simulink model "wlan_phy_rx_pmd"  (Simulink 7.8)
settings: Solver=VariableStepDiscrete  SolverMode=SingleTasking  StartTime=0.0  StopTime=rx_sim.sim_time  RelTol=1e-3
PostLoadFcn:
  | wlan_phy_rx_init
InitFcn:
  | wlan_phy_rx_init
StartFcn:
  | wlan_phy_rx_init
values from neighbouring files (symbols this model uses):
  barker_seq20 = 0.95 * circshift(barker_seq20, [0 4]) ./ max(abs(barker_seq20))    [wlan_phy_rx_init.m]
  bit_scrambler_lfsr_bytes = sum(bit_scrambler_lfsr_bytes .* repmat(2.^[0:7], 127, 1),2)    [wlan_phy_rx_init.m]
  longCorr_coef_i = [3*real(longCorr_coef)]    [wlan_phy_rx_init.m]
  longCorr_coef_q = [3*imag(longCorr_coef)]    [wlan_phy_rx_init.m]
  MAX_CP_LEN = 32    [wlan_phy_rx_init.m]
  MAX_NCBPS = NCBPS_11n_64QAM    (= 312)    [wlan_phy_rx_init.m]
  MAX_NUM_BYTES = 2^16-1    (= 65535)    [wlan_phy_rx_init.m]
  MAX_NUM_SC = 64    [wlan_phy_rx_init.m]
  MAX_NUM_SYMS = 600    [wlan_phy_rx_init.m]
  NDBPS_11a_BPSK12 = 24    [wlan_phy_rx_init.m]
  preFFT_sampBuff_numSamps = 4*MAX_NUM_SC    (= 256)    [wlan_phy_rx_init.m]
  rx_sim = struct()    [wlan_phy_rx_init.m]
  scr_ind_rev = zeros(1,128)    [wlan_phy_rx_init.m]
undefined symbols (not defined in the file or in its folder): gcbh, mcs_rom_11ag, mcs_rom_11n, wlan_rx_deinterleave_rom_gen, xlBlockLoadCallback, xlGetNormalizedPeriod, xlipgetpartsetting
libraries: xbsIndex_r4 x4943, xbsEDKLib_r4 x1
(part 21 of 28)

== system wlan_phy_rx_pmd/Sync & Antenna Sel/LTS Correlation/Correlator/C4/C4 ==
  x : Inport
  vin : Inport  Port=2
  "  " : Inport  Port=3
  "     " : Inport  Port=4
  ASR : xbsIndex_r4/Addressable Shift Register  infer_latency=off  depth=length(coef2)  initVector=[0]  en=on  dbl_ovrd=off  use_behavioral_HDL=off  Optimization=Resource  use_rpm=off  block_type=addrsr
      settings: infoedit="Delay of configurable length.  Any element in the delay line can be addressed and driven onto the output port.<br><br>Hardware notes: This block is implemented using SRL16s. If Virtex-4 or Spartan-3 device is used, multiple SRLC16s are cascaded together."  xl_area=[1 0 0 2 0 0 0]  block_version=VER_STRING_GOES_HERE
  Delay : xbsIndex_r4/Delay  rst=off  infoeditControl=Selection of Reset will increase slice count due to use of real FFs and instead of SRLs  en=off  latency=1  dbl_ovrd=off  reg_retiming=off  block_type=delay
  Gateway Out{1..8} (8 alike) : xbsIndex_r4/Gateway Out  inherit_from_input=off  hdl_port=off  timing_constraint=None  locs_specified=off  LOCs={}  UseAsDAC=off  DACChannel='1'  block_type=gatewayout
      settings: infoedit="Gateway out block.  Converts Xilinx fixed point inputs into ouputs of type Simulink integer, double, or fixed point.<P><P>Hardware notes:  In hardware these blocks become top level output ports or are discarded, depending on how they are configured."  xl_area=[0 0 0 0 0 0 0]  block_version=VER_STRING_GOES_HERE
  MAC : Subsystem
  ROM : xbsIndex_r4/ROM  depth=length(coef2)  initVector=coef2  distributed_mem=Distributed memory  rst=off  init_reg=0  en=off  latency=1  gui_display_data_type=Fixed-point  arith_type="Signed  (2's comp)"  n_bits=longCorr_coef_nbits  bin_pt=longCorr_coef_bp  preci_type=Single  exp_width=8  frac_width=24  dbl_ovrd=off  optimize=Area  use_rpm=off  block_type=sprom
      settings: xl_area=[1 1 0 1 0 0 0]  block_version=VER_STRING_GOES_HERE
  x_dly : Outport
  x_dly_vout : Outport  Port=2
  y : Outport  Port=3
  y_vout : Outport  Port=4
  wires:
  Gateway Out5/1 -> ?  "ROM Addr"
  Gateway Out4/1 -> ?  "Corr Out"
  Gateway Out3/1 -> ?  "Corr Ld"
  Gateway Out2/1 -> ?  "ROM Out"
  Gateway Out1/1 -> ?  "ASR Out"
  Gateway Out6/1 -> ?  "X In"
  Gateway Out7/1 -> ?  "ASR Addr"
  Gateway Out8/1 -> ?  "X Vin"
  Delay/1 -> y_vout/1
  vin/1 -> x_dly_vout/1, MAC/4(rst), Gateway Out8/1, ASR/3
  x/1 -> Gateway Out6/1, ASR/1
  [ltsCorr_dAddr] -> Gateway Out7/1, ASR/2
  [ltsCorr_cAddr] -> Gateway Out5/1, ROM/1
  ASR/1 -> Gateway Out1/1, x_dly/1, MAC/1(a)
  MAC/1(yn) -> Gateway Out4/1, y/1
  ROM/1 -> Gateway Out2/1, MAC/2(coeff)
  [ltsCorr_ld] -> Gateway Out3/1, Delay/1, MAC/3(ld)

== system wlan_phy_rx_pmd/Sync & Antenna Sel/LTS Correlation/Correlator/C4/C5 ==
  x : Inport
  vin : Inport  Port=2
  "  " : Inport  Port=3
  "     " : Inport  Port=4
  ASR : xbsIndex_r4/Addressable Shift Register  infer_latency=off  depth=length(coef2)  initVector=[0]  en=on  dbl_ovrd=off  use_behavioral_HDL=off  Optimization=Resource  use_rpm=off  block_type=addrsr
      settings: infoedit="Delay of configurable length.  Any element in the delay line can be addressed and driven onto the output port.<br><br>Hardware notes: This block is implemented using SRL16s. If Virtex-4 or Spartan-3 device is used, multiple SRLC16s are cascaded together."  xl_area=[1 0 0 2 0 0 0]  block_version=VER_STRING_GOES_HERE
  Delay : xbsIndex_r4/Delay  rst=off  infoeditControl=Selection of Reset will increase slice count due to use of real FFs and instead of SRLs  en=off  latency=1  dbl_ovrd=off  reg_retiming=off  block_type=delay
  Gateway Out{1..8} (8 alike) : xbsIndex_r4/Gateway Out  inherit_from_input=off  hdl_port=off  timing_constraint=None  locs_specified=off  LOCs={}  UseAsDAC=off  DACChannel='1'  block_type=gatewayout
      settings: infoedit="Gateway out block.  Converts Xilinx fixed point inputs into ouputs of type Simulink integer, double, or fixed point.<P><P>Hardware notes:  In hardware these blocks become top level output ports or are discarded, depending on how they are configured."  xl_area=[0 0 0 0 0 0 0]  block_version=VER_STRING_GOES_HERE
  MAC : Subsystem
  ROM : xbsIndex_r4/ROM  depth=length(coef2)  initVector=coef2  distributed_mem=Distributed memory  rst=off  init_reg=0  en=off  latency=1  gui_display_data_type=Fixed-point  arith_type="Signed  (2's comp)"  n_bits=longCorr_coef_nbits  bin_pt=longCorr_coef_bp  preci_type=Single  exp_width=8  frac_width=24  dbl_ovrd=off  optimize=Area  use_rpm=off  block_type=sprom
      settings: xl_area=[1 1 0 1 0 0 0]  block_version=VER_STRING_GOES_HERE
  x_dly : Outport
  x_dly_vout : Outport  Port=2
  y : Outport  Port=3
  y_vout : Outport  Port=4
  wires:
  [ltsCorr_ld] -> MAC/3(ld), Delay/1, Gateway Out3/1
  ROM/1 -> MAC/2(coeff), Gateway Out2/1
  MAC/1(yn) -> y/1, Gateway Out4/1
  ASR/1 -> MAC/1(a), x_dly/1, Gateway Out1/1
  [ltsCorr_cAddr] -> ROM/1, Gateway Out5/1
  [ltsCorr_dAddr] -> ASR/2, Gateway Out7/1
  x/1 -> ASR/1, Gateway Out6/1
  vin/1 -> ASR/3, Gateway Out8/1, MAC/4(rst), x_dly_vout/1
  Delay/1 -> y_vout/1
  Gateway Out8/1 -> ?  "X Vin"
  Gateway Out7/1 -> ?  "ASR Addr"
  Gateway Out6/1 -> ?  "X In"
  Gateway Out1/1 -> ?  "ASR Out"
  Gateway Out2/1 -> ?  "ROM Out"
  Gateway Out3/1 -> ?  "Corr Ld"
  Gateway Out4/1 -> ?  "Corr Out"
  Gateway Out5/1 -> ?  "ROM Addr"

== system wlan_phy_rx_pmd/Sync & Antenna Sel/LTS Correlation/Correlator/C4/C6 ==
  x : Inport
  vin : Inport  Port=2
  "  " : Inport  Port=3
  "     " : Inport  Port=4
  ASR : xbsIndex_r4/Addressable Shift Register  infer_latency=off  depth=length(coef2)  initVector=[0]  en=on  dbl_ovrd=off  use_behavioral_HDL=off  Optimization=Resource  use_rpm=off  block_type=addrsr
      settings: infoedit="Delay of configurable length.  Any element in the delay line can be addressed and driven onto the output port.<br><br>Hardware notes: This block is implemented using SRL16s. If Virtex-4 or Spartan-3 device is used, multiple SRLC16s are cascaded together."  xl_area=[1 0 0 2 0 0 0]  block_version=VER_STRING_GOES_HERE
  Delay : xbsIndex_r4/Delay  rst=off  infoeditControl=Selection of Reset will increase slice count due to use of real FFs and instead of SRLs  en=off  latency=1  dbl_ovrd=off  reg_retiming=off  block_type=delay
  Gateway Out{1..8} (8 alike) : xbsIndex_r4/Gateway Out  inherit_from_input=off  hdl_port=off  timing_constraint=None  locs_specified=off  LOCs={}  UseAsDAC=off  DACChannel='1'  block_type=gatewayout
      settings: infoedit="Gateway out block.  Converts Xilinx fixed point inputs into ouputs of type Simulink integer, double, or fixed point.<P><P>Hardware notes:  In hardware these blocks become top level output ports or are discarded, depending on how they are configured."  xl_area=[0 0 0 0 0 0 0]  block_version=VER_STRING_GOES_HERE
  MAC : Subsystem
  ROM : xbsIndex_r4/ROM  depth=length(coef2)  initVector=coef2  distributed_mem=Distributed memory  rst=off  init_reg=0  en=off  latency=1  gui_display_data_type=Fixed-point  arith_type="Signed  (2's comp)"  n_bits=longCorr_coef_nbits  bin_pt=longCorr_coef_bp  preci_type=Single  exp_width=8  frac_width=24  dbl_ovrd=off  optimize=Area  use_rpm=off  block_type=sprom
      settings: xl_area=[1 1 0 1 0 0 0]  block_version=VER_STRING_GOES_HERE
  x_dly : Outport
  x_dly_vout : Outport  Port=2
  y : Outport  Port=3
  y_vout : Outport  Port=4
  wires:
  Gateway Out5/1 -> ?  "ROM Addr"
  Gateway Out4/1 -> ?  "Corr Out"
  Gateway Out3/1 -> ?  "Corr Ld"
  Gateway Out2/1 -> ?  "ROM Out"
  Gateway Out1/1 -> ?  "ASR Out"
  Gateway Out6/1 -> ?  "X In"
  Gateway Out7/1 -> ?  "ASR Addr"
  Gateway Out8/1 -> ?  "X Vin"
  Delay/1 -> y_vout/1
  vin/1 -> x_dly_vout/1, MAC/4(rst), Gateway Out8/1, ASR/3
  x/1 -> Gateway Out6/1, ASR/1
  [ltsCorr_dAddr] -> Gateway Out7/1, ASR/2
  [ltsCorr_cAddr] -> Gateway Out5/1, ROM/1
  ASR/1 -> Gateway Out1/1, x_dly/1, MAC/1(a)
  MAC/1(yn) -> Gateway Out4/1, y/1
  ROM/1 -> Gateway Out2/1, MAC/2(coeff)
  [ltsCorr_ld] -> Gateway Out3/1, Delay/1, MAC/3(ld)

== system wlan_phy_rx_pmd/Sync & Antenna Sel/LTS Correlation/Correlator/C4/C7 ==
  x : Inport
  vin : Inport  Port=2
  "  " : Inport  Port=3
  "     " : Inport  Port=4
  ASR : xbsIndex_r4/Addressable Shift Register  infer_latency=off  depth=length(coef2)  initVector=[0]  en=on  dbl_ovrd=off  use_behavioral_HDL=off  Optimization=Resource  use_rpm=off  block_type=addrsr
      settings: infoedit="Delay of configurable length.  Any element in the delay line can be addressed and driven onto the output port.<br><br>Hardware notes: This block is implemented using SRL16s. If Virtex-4 or Spartan-3 device is used, multiple SRLC16s are cascaded together."  xl_area=[1 0 0 2 0 0 0]  block_version=VER_STRING_GOES_HERE
  Delay : xbsIndex_r4/Delay  rst=off  infoeditControl=Selection of Reset will increase slice count due to use of real FFs and instead of SRLs  en=off  latency=1  dbl_ovrd=off  reg_retiming=off  block_type=delay
  Gateway Out{1..8} (8 alike) : xbsIndex_r4/Gateway Out  inherit_from_input=off  hdl_port=off  timing_constraint=None  locs_specified=off  LOCs={}  UseAsDAC=off  DACChannel='1'  block_type=gatewayout
      settings: infoedit="Gateway out block.  Converts Xilinx fixed point inputs into ouputs of type Simulink integer, double, or fixed point.<P><P>Hardware notes:  In hardware these blocks become top level output ports or are discarded, depending on how they are configured."  xl_area=[0 0 0 0 0 0 0]  block_version=VER_STRING_GOES_HERE
  MAC : Subsystem
  ROM : xbsIndex_r4/ROM  depth=length(coef2)  initVector=coef2  distributed_mem=Distributed memory  rst=off  init_reg=0  en=off  latency=1  gui_display_data_type=Fixed-point  arith_type="Signed  (2's comp)"  n_bits=longCorr_coef_nbits  bin_pt=longCorr_coef_bp  preci_type=Single  exp_width=8  frac_width=24  dbl_ovrd=off  optimize=Area  use_rpm=off  block_type=sprom
      settings: xl_area=[1 1 0 1 0 0 0]  block_version=VER_STRING_GOES_HERE
  x_dly : Outport
  x_dly_vout : Outport  Port=2
  y : Outport  Port=3
  y_vout : Outport  Port=4
  wires:
  [ltsCorr_ld] -> MAC/3(ld), Delay/1, Gateway Out3/1
  ROM/1 -> MAC/2(coeff), Gateway Out2/1
  MAC/1(yn) -> y/1, Gateway Out4/1
  ASR/1 -> MAC/1(a), x_dly/1, Gateway Out1/1
  [ltsCorr_cAddr] -> ROM/1, Gateway Out5/1
  [ltsCorr_dAddr] -> ASR/2, Gateway Out7/1
  x/1 -> ASR/1, Gateway Out6/1
  vin/1 -> ASR/3, Gateway Out8/1, MAC/4(rst), x_dly_vout/1
  Delay/1 -> y_vout/1
  Gateway Out8/1 -> ?  "X Vin"
  Gateway Out7/1 -> ?  "ASR Addr"
  Gateway Out6/1 -> ?  "X In"
  Gateway Out1/1 -> ?  "ASR Out"
  Gateway Out2/1 -> ?  "ROM Out"
  Gateway Out3/1 -> ?  "Corr Ld"
  Gateway Out4/1 -> ?  "Corr Out"
  Gateway Out5/1 -> ?  "ROM Addr"

== system wlan_phy_rx_pmd/Sync & Antenna Sel/LTS Correlation/Correlator/C4/C8 ==
  x : Inport
  vin : Inport  Port=2
  "  " : Inport  Port=3
  "     " : Inport  Port=4
  ASR : xbsIndex_r4/Addressable Shift Register  infer_latency=off  depth=length(coef2)  initVector=[0]  en=on  dbl_ovrd=off  use_behavioral_HDL=off  Optimization=Resource  use_rpm=off  block_type=addrsr
      settings: infoedit="Delay of configurable length.  Any element in the delay line can be addressed and driven onto the output port.<br><br>Hardware notes: This block is implemented using SRL16s. If Virtex-4 or Spartan-3 device is used, multiple SRLC16s are cascaded together."  xl_area=[1 0 0 2 0 0 0]  block_version=VER_STRING_GOES_HERE
  Delay : xbsIndex_r4/Delay  rst=off  infoeditControl=Selection of Reset will increase slice count due to use of real FFs and instead of SRLs  en=off  latency=1  dbl_ovrd=off  reg_retiming=off  block_type=delay
  Gateway Out{1..8} (8 alike) : xbsIndex_r4/Gateway Out  inherit_from_input=off  hdl_port=off  timing_constraint=None  locs_specified=off  LOCs={}  UseAsDAC=off  DACChannel='1'  block_type=gatewayout
      settings: infoedit="Gateway out block.  Converts Xilinx fixed point inputs into ouputs of type Simulink integer, double, or fixed point.<P><P>Hardware notes:  In hardware these blocks become top level output ports or are discarded, depending on how they are configured."  xl_area=[0 0 0 0 0 0 0]  block_version=VER_STRING_GOES_HERE
  MAC : Subsystem
  ROM : xbsIndex_r4/ROM  depth=length(coef2)  initVector=coef2  distributed_mem=Distributed memory  rst=off  init_reg=0  en=off  latency=1  gui_display_data_type=Fixed-point  arith_type="Signed  (2's comp)"  n_bits=longCorr_coef_nbits  bin_pt=longCorr_coef_bp  preci_type=Single  exp_width=8  frac_width=24  dbl_ovrd=off  optimize=Area  use_rpm=off  block_type=sprom
      settings: xl_area=[1 1 0 1 0 0 0]  block_version=VER_STRING_GOES_HERE
  x_dly : Outport
  x_dly_vout : Outport  Port=2
  y : Outport  Port=3
  y_vout : Outport  Port=4
  wires:
  Gateway Out5/1 -> ?  "ROM Addr"
  Gateway Out4/1 -> ?  "Corr Out"
  Gateway Out3/1 -> ?  "Corr Ld"
  Gateway Out2/1 -> ?  "ROM Out"
  Gateway Out1/1 -> ?  "ASR Out"
  Gateway Out6/1 -> ?  "X In"
  Gateway Out7/1 -> ?  "ASR Addr"
  Gateway Out8/1 -> ?  "X Vin"
  Delay/1 -> y_vout/1
  vin/1 -> x_dly_vout/1, MAC/4(rst), Gateway Out8/1, ASR/3
  x/1 -> Gateway Out6/1, ASR/1
  [ltsCorr_dAddr] -> Gateway Out7/1, ASR/2
  [ltsCorr_cAddr] -> Gateway Out5/1, ROM/1
  ASR/1 -> Gateway Out1/1, x_dly/1, MAC/1(a)
  MAC/1(yn) -> Gateway Out4/1, y/1
  ROM/1 -> Gateway Out2/1, MAC/2(coeff)
  [ltsCorr_ld] -> Gateway Out3/1, Delay/1, MAC/3(ld)

== system wlan_phy_rx_pmd/Sync & Antenna Sel/LTS Correlation/Correlator/C5/C1 ==
  x : Inport
  vin : Inport  Port=2
  "  " : Inport  Port=3
  "     " : Inport  Port=4
  ASR : xbsIndex_r4/Addressable Shift Register  infer_latency=off  depth=length(coef2)  initVector=[0]  en=on  dbl_ovrd=off  use_behavioral_HDL=off  Optimization=Resource  use_rpm=off  block_type=addrsr
      settings: infoedit="Delay of configurable length.  Any element in the delay line can be addressed and driven onto the output port.<br><br>Hardware notes: This block is implemented using SRL16s. If Virtex-4 or Spartan-3 device is used, multiple SRLC16s are cascaded together."  xl_area=[1 0 0 2 0 0 0]  block_version=VER_STRING_GOES_HERE
  Delay : xbsIndex_r4/Delay  rst=off  infoeditControl=Selection of Reset will increase slice count due to use of real FFs and instead of SRLs  en=off  latency=1  dbl_ovrd=off  reg_retiming=off  block_type=delay
  Gateway Out{1..8} (8 alike) : xbsIndex_r4/Gateway Out  inherit_from_input=off  hdl_port=off  timing_constraint=None  locs_specified=off  LOCs={}  UseAsDAC=off  DACChannel='1'  block_type=gatewayout
      settings: infoedit="Gateway out block.  Converts Xilinx fixed point inputs into ouputs of type Simulink integer, double, or fixed point.<P><P>Hardware notes:  In hardware these blocks become top level output ports or are discarded, depending on how they are configured."  xl_area=[0 0 0 0 0 0 0]  block_version=VER_STRING_GOES_HERE
  MAC : Subsystem
  ROM : xbsIndex_r4/ROM  depth=length(coef2)  initVector=coef2  distributed_mem=Distributed memory  rst=off  init_reg=0  en=off  latency=1  gui_display_data_type=Fixed-point  arith_type="Signed  (2's comp)"  n_bits=longCorr_coef_nbits  bin_pt=longCorr_coef_bp  preci_type=Single  exp_width=8  frac_width=24  dbl_ovrd=off  optimize=Area  use_rpm=off  block_type=sprom
      settings: xl_area=[1 1 0 1 0 0 0]  block_version=VER_STRING_GOES_HERE
  x_dly : Outport
  x_dly_vout : Outport  Port=2
  y : Outport  Port=3
  y_vout : Outport  Port=4
  wires:
  Gateway Out5/1 -> ?  "ROM Addr"
  Gateway Out4/1 -> ?  "Corr Out"
  Gateway Out3/1 -> ?  "Corr Ld"
  Gateway Out2/1 -> ?  "ROM Out"
  Gateway Out1/1 -> ?  "ASR Out"
  Gateway Out6/1 -> ?  "X In"
  Gateway Out7/1 -> ?  "ASR Addr"
  Gateway Out8/1 -> ?  "X Vin"
  Delay/1 -> y_vout/1
  vin/1 -> x_dly_vout/1, MAC/4(rst), Gateway Out8/1, ASR/3
  x/1 -> Gateway Out6/1, ASR/1
  [ltsCorr_dAddr] -> Gateway Out7/1, ASR/2
  [ltsCorr_cAddr] -> Gateway Out5/1, ROM/1
  ASR/1 -> Gateway Out1/1, x_dly/1, MAC/1(a)
  MAC/1(yn) -> Gateway Out4/1, y/1
  ROM/1 -> Gateway Out2/1, MAC/2(coeff)
  [ltsCorr_ld] -> Gateway Out3/1, Delay/1, MAC/3(ld)

== system wlan_phy_rx_pmd/Sync & Antenna Sel/LTS Correlation/Correlator/C5/C2 ==
  x : Inport
  vin : Inport  Port=2
  "  " : Inport  Port=3
  "     " : Inport  Port=4
  ASR : xbsIndex_r4/Addressable Shift Register  infer_latency=off  depth=length(coef2)  initVector=[0]  en=on  dbl_ovrd=off  use_behavioral_HDL=off  Optimization=Resource  use_rpm=off  block_type=addrsr
      settings: infoedit="Delay of configurable length.  Any element in the delay line can be addressed and driven onto the output port.<br><br>Hardware notes: This block is implemented using SRL16s. If Virtex-4 or Spartan-3 device is used, multiple SRLC16s are cascaded together."  xl_area=[1 0 0 2 0 0 0]  block_version=VER_STRING_GOES_HERE
  Delay : xbsIndex_r4/Delay  rst=off  infoeditControl=Selection of Reset will increase slice count due to use of real FFs and instead of SRLs  en=off  latency=1  dbl_ovrd=off  reg_retiming=off  block_type=delay
  Gateway Out{1..8} (8 alike) : xbsIndex_r4/Gateway Out  inherit_from_input=off  hdl_port=off  timing_constraint=None  locs_specified=off  LOCs={}  UseAsDAC=off  DACChannel='1'  block_type=gatewayout
      settings: infoedit="Gateway out block.  Converts Xilinx fixed point inputs into ouputs of type Simulink integer, double, or fixed point.<P><P>Hardware notes:  In hardware these blocks become top level output ports or are discarded, depending on how they are configured."  xl_area=[0 0 0 0 0 0 0]  block_version=VER_STRING_GOES_HERE
  MAC : Subsystem
  ROM : xbsIndex_r4/ROM  depth=length(coef2)  initVector=coef2  distributed_mem=Distributed memory  rst=off  init_reg=0  en=off  latency=1  gui_display_data_type=Fixed-point  arith_type="Signed  (2's comp)"  n_bits=longCorr_coef_nbits  bin_pt=longCorr_coef_bp  preci_type=Single  exp_width=8  frac_width=24  dbl_ovrd=off  optimize=Area  use_rpm=off  block_type=sprom
      settings: xl_area=[1 1 0 1 0 0 0]  block_version=VER_STRING_GOES_HERE
  x_dly : Outport
  x_dly_vout : Outport  Port=2
  y : Outport  Port=3
  y_vout : Outport  Port=4
  wires:
  [ltsCorr_ld] -> MAC/3(ld), Delay/1, Gateway Out3/1
  ROM/1 -> MAC/2(coeff), Gateway Out2/1
  MAC/1(yn) -> y/1, Gateway Out4/1
  ASR/1 -> MAC/1(a), x_dly/1, Gateway Out1/1
  [ltsCorr_cAddr] -> ROM/1, Gateway Out5/1
  [ltsCorr_dAddr] -> ASR/2, Gateway Out7/1
  x/1 -> ASR/1, Gateway Out6/1
  vin/1 -> ASR/3, Gateway Out8/1, MAC/4(rst), x_dly_vout/1
  Delay/1 -> y_vout/1
  Gateway Out8/1 -> ?  "X Vin"
  Gateway Out7/1 -> ?  "ASR Addr"
  Gateway Out6/1 -> ?  "X In"
  Gateway Out1/1 -> ?  "ASR Out"
  Gateway Out2/1 -> ?  "ROM Out"
  Gateway Out3/1 -> ?  "Corr Ld"
  Gateway Out4/1 -> ?  "Corr Out"
  Gateway Out5/1 -> ?  "ROM Addr"

== system wlan_phy_rx_pmd/Sync & Antenna Sel/LTS Correlation/Correlator/C5/C3 ==
  x : Inport
  vin : Inport  Port=2
  "  " : Inport  Port=3
  "     " : Inport  Port=4
  ASR : xbsIndex_r4/Addressable Shift Register  infer_latency=off  depth=length(coef2)  initVector=[0]  en=on  dbl_ovrd=off  use_behavioral_HDL=off  Optimization=Resource  use_rpm=off  block_type=addrsr
      settings: infoedit="Delay of configurable length.  Any element in the delay line can be addressed and driven onto the output port.<br><br>Hardware notes: This block is implemented using SRL16s. If Virtex-4 or Spartan-3 device is used, multiple SRLC16s are cascaded together."  xl_area=[1 0 0 2 0 0 0]  block_version=VER_STRING_GOES_HERE
  Delay : xbsIndex_r4/Delay  rst=off  infoeditControl=Selection of Reset will increase slice count due to use of real FFs and instead of SRLs  en=off  latency=1  dbl_ovrd=off  reg_retiming=off  block_type=delay
  Gateway Out{1..8} (8 alike) : xbsIndex_r4/Gateway Out  inherit_from_input=off  hdl_port=off  timing_constraint=None  locs_specified=off  LOCs={}  UseAsDAC=off  DACChannel='1'  block_type=gatewayout
      settings: infoedit="Gateway out block.  Converts Xilinx fixed point inputs into ouputs of type Simulink integer, double, or fixed point.<P><P>Hardware notes:  In hardware these blocks become top level output ports or are discarded, depending on how they are configured."  xl_area=[0 0 0 0 0 0 0]  block_version=VER_STRING_GOES_HERE
  MAC : Subsystem
  ROM : xbsIndex_r4/ROM  depth=length(coef2)  initVector=coef2  distributed_mem=Distributed memory  rst=off  init_reg=0  en=off  latency=1  gui_display_data_type=Fixed-point  arith_type="Signed  (2's comp)"  n_bits=longCorr_coef_nbits  bin_pt=longCorr_coef_bp  preci_type=Single  exp_width=8  frac_width=24  dbl_ovrd=off  optimize=Area  use_rpm=off  block_type=sprom
      settings: xl_area=[1 1 0 1 0 0 0]  block_version=VER_STRING_GOES_HERE
  x_dly : Outport
  x_dly_vout : Outport  Port=2
  y : Outport  Port=3
  y_vout : Outport  Port=4
  wires:
  Gateway Out5/1 -> ?  "ROM Addr"
  Gateway Out4/1 -> ?  "Corr Out"
  Gateway Out3/1 -> ?  "Corr Ld"
  Gateway Out2/1 -> ?  "ROM Out"
  Gateway Out1/1 -> ?  "ASR Out"
  Gateway Out6/1 -> ?  "X In"
  Gateway Out7/1 -> ?  "ASR Addr"
  Gateway Out8/1 -> ?  "X Vin"
  Delay/1 -> y_vout/1
  vin/1 -> x_dly_vout/1, MAC/4(rst), Gateway Out8/1, ASR/3
  x/1 -> Gateway Out6/1, ASR/1
  [ltsCorr_dAddr] -> Gateway Out7/1, ASR/2
  [ltsCorr_cAddr] -> Gateway Out5/1, ROM/1
  ASR/1 -> Gateway Out1/1, x_dly/1, MAC/1(a)
  MAC/1(yn) -> Gateway Out4/1, y/1
  ROM/1 -> Gateway Out2/1, MAC/2(coeff)
  [ltsCorr_ld] -> Gateway Out3/1, Delay/1, MAC/3(ld)

== system wlan_phy_rx_pmd/Sync & Antenna Sel/LTS Correlation/Correlator/C5/C4 ==
  x : Inport
  vin : Inport  Port=2
  "  " : Inport  Port=3
  "     " : Inport  Port=4
  ASR : xbsIndex_r4/Addressable Shift Register  infer_latency=off  depth=length(coef2)  initVector=[0]  en=on  dbl_ovrd=off  use_behavioral_HDL=off  Optimization=Resource  use_rpm=off  block_type=addrsr
      settings: infoedit="Delay of configurable length.  Any element in the delay line can be addressed and driven onto the output port.<br><br>Hardware notes: This block is implemented using SRL16s. If Virtex-4 or Spartan-3 device is used, multiple SRLC16s are cascaded together."  xl_area=[1 0 0 2 0 0 0]  block_version=VER_STRING_GOES_HERE
  Delay : xbsIndex_r4/Delay  rst=off  infoeditControl=Selection of Reset will increase slice count due to use of real FFs and instead of SRLs  en=off  latency=1  dbl_ovrd=off  reg_retiming=off  block_type=delay
  Gateway Out{1..8} (8 alike) : xbsIndex_r4/Gateway Out  inherit_from_input=off  hdl_port=off  timing_constraint=None  locs_specified=off  LOCs={}  UseAsDAC=off  DACChannel='1'  block_type=gatewayout
      settings: infoedit="Gateway out block.  Converts Xilinx fixed point inputs into ouputs of type Simulink integer, double, or fixed point.<P><P>Hardware notes:  In hardware these blocks become top level output ports or are discarded, depending on how they are configured."  xl_area=[0 0 0 0 0 0 0]  block_version=VER_STRING_GOES_HERE
  MAC : Subsystem
  ROM : xbsIndex_r4/ROM  depth=length(coef2)  initVector=coef2  distributed_mem=Distributed memory  rst=off  init_reg=0  en=off  latency=1  gui_display_data_type=Fixed-point  arith_type="Signed  (2's comp)"  n_bits=longCorr_coef_nbits  bin_pt=longCorr_coef_bp  preci_type=Single  exp_width=8  frac_width=24  dbl_ovrd=off  optimize=Area  use_rpm=off  block_type=sprom
      settings: xl_area=[1 1 0 1 0 0 0]  block_version=VER_STRING_GOES_HERE
  x_dly : Outport
  x_dly_vout : Outport  Port=2
  y : Outport  Port=3
  y_vout : Outport  Port=4
  wires:
  [ltsCorr_ld] -> MAC/3(ld), Delay/1, Gateway Out3/1
  ROM/1 -> MAC/2(coeff), Gateway Out2/1
  MAC/1(yn) -> y/1, Gateway Out4/1
  ASR/1 -> MAC/1(a), x_dly/1, Gateway Out1/1
  [ltsCorr_cAddr] -> ROM/1, Gateway Out5/1
  [ltsCorr_dAddr] -> ASR/2, Gateway Out7/1
  x/1 -> ASR/1, Gateway Out6/1
  vin/1 -> ASR/3, Gateway Out8/1, MAC/4(rst), x_dly_vout/1
  Delay/1 -> y_vout/1
  Gateway Out8/1 -> ?  "X Vin"
  Gateway Out7/1 -> ?  "ASR Addr"
  Gateway Out6/1 -> ?  "X In"
  Gateway Out1/1 -> ?  "ASR Out"
  Gateway Out2/1 -> ?  "ROM Out"
  Gateway Out3/1 -> ?  "Corr Ld"
  Gateway Out4/1 -> ?  "Corr Out"
  Gateway Out5/1 -> ?  "ROM Addr"

== system wlan_phy_rx_pmd/Sync & Antenna Sel/LTS Correlation/Correlator/C5/C5 ==
  x : Inport
  vin : Inport  Port=2
  "  " : Inport  Port=3
  "     " : Inport  Port=4
  ASR : xbsIndex_r4/Addressable Shift Register  infer_latency=off  depth=length(coef2)  initVector=[0]  en=on  dbl_ovrd=off  use_behavioral_HDL=off  Optimization=Resource  use_rpm=off  block_type=addrsr
      settings: infoedit="Delay of configurable length.  Any element in the delay line can be addressed and driven onto the output port.<br><br>Hardware notes: This block is implemented using SRL16s. If Virtex-4 or Spartan-3 device is used, multiple SRLC16s are cascaded together."  xl_area=[1 0 0 2 0 0 0]  block_version=VER_STRING_GOES_HERE
  Delay : xbsIndex_r4/Delay  rst=off  infoeditControl=Selection of Reset will increase slice count due to use of real FFs and instead of SRLs  en=off  latency=1  dbl_ovrd=off  reg_retiming=off  block_type=delay
  Gateway Out{1..8} (8 alike) : xbsIndex_r4/Gateway Out  inherit_from_input=off  hdl_port=off  timing_constraint=None  locs_specified=off  LOCs={}  UseAsDAC=off  DACChannel='1'  block_type=gatewayout
      settings: infoedit="Gateway out block.  Converts Xilinx fixed point inputs into ouputs of type Simulink integer, double, or fixed point.<P><P>Hardware notes:  In hardware these blocks become top level output ports or are discarded, depending on how they are configured."  xl_area=[0 0 0 0 0 0 0]  block_version=VER_STRING_GOES_HERE
  MAC : Subsystem
  ROM : xbsIndex_r4/ROM  depth=length(coef2)  initVector=coef2  distributed_mem=Distributed memory  rst=off  init_reg=0  en=off  latency=1  gui_display_data_type=Fixed-point  arith_type="Signed  (2's comp)"  n_bits=longCorr_coef_nbits  bin_pt=longCorr_coef_bp  preci_type=Single  exp_width=8  frac_width=24  dbl_ovrd=off  optimize=Area  use_rpm=off  block_type=sprom
      settings: xl_area=[1 1 0 1 0 0 0]  block_version=VER_STRING_GOES_HERE
  x_dly : Outport
  x_dly_vout : Outport  Port=2
  y : Outport  Port=3
  y_vout : Outport  Port=4
  wires:
  Gateway Out5/1 -> ?  "ROM Addr"
  Gateway Out4/1 -> ?  "Corr Out"
  Gateway Out3/1 -> ?  "Corr Ld"
  Gateway Out2/1 -> ?  "ROM Out"
  Gateway Out1/1 -> ?  "ASR Out"
  Gateway Out6/1 -> ?  "X In"
  Gateway Out7/1 -> ?  "ASR Addr"
  Gateway Out8/1 -> ?  "X Vin"
  Delay/1 -> y_vout/1
  vin/1 -> x_dly_vout/1, MAC/4(rst), Gateway Out8/1, ASR/3
  x/1 -> Gateway Out6/1, ASR/1
  [ltsCorr_dAddr] -> Gateway Out7/1, ASR/2
  [ltsCorr_cAddr] -> Gateway Out5/1, ROM/1
  ASR/1 -> Gateway Out1/1, x_dly/1, MAC/1(a)
  MAC/1(yn) -> Gateway Out4/1, y/1
  ROM/1 -> Gateway Out2/1, MAC/2(coeff)
  [ltsCorr_ld] -> Gateway Out3/1, Delay/1, MAC/3(ld)

== system wlan_phy_rx_pmd/Sync & Antenna Sel/LTS Correlation/Correlator/C5/C6 ==
  x : Inport
  vin : Inport  Port=2
  "  " : Inport  Port=3
  "     " : Inport  Port=4
  ASR : xbsIndex_r4/Addressable Shift Register  infer_latency=off  depth=length(coef2)  initVector=[0]  en=on  dbl_ovrd=off  use_behavioral_HDL=off  Optimization=Resource  use_rpm=off  block_type=addrsr
      settings: infoedit="Delay of configurable length.  Any element in the delay line can be addressed and driven onto the output port.<br><br>Hardware notes: This block is implemented using SRL16s. If Virtex-4 or Spartan-3 device is used, multiple SRLC16s are cascaded together."  xl_area=[1 0 0 2 0 0 0]  block_version=VER_STRING_GOES_HERE
  Delay : xbsIndex_r4/Delay  rst=off  infoeditControl=Selection of Reset will increase slice count due to use of real FFs and instead of SRLs  en=off  latency=1  dbl_ovrd=off  reg_retiming=off  block_type=delay
  Gateway Out{1..8} (8 alike) : xbsIndex_r4/Gateway Out  inherit_from_input=off  hdl_port=off  timing_constraint=None  locs_specified=off  LOCs={}  UseAsDAC=off  DACChannel='1'  block_type=gatewayout
      settings: infoedit="Gateway out block.  Converts Xilinx fixed point inputs into ouputs of type Simulink integer, double, or fixed point.<P><P>Hardware notes:  In hardware these blocks become top level output ports or are discarded, depending on how they are configured."  xl_area=[0 0 0 0 0 0 0]  block_version=VER_STRING_GOES_HERE
  MAC : Subsystem
  ROM : xbsIndex_r4/ROM  depth=length(coef2)  initVector=coef2  distributed_mem=Distributed memory  rst=off  init_reg=0  en=off  latency=1  gui_display_data_type=Fixed-point  arith_type="Signed  (2's comp)"  n_bits=longCorr_coef_nbits  bin_pt=longCorr_coef_bp  preci_type=Single  exp_width=8  frac_width=24  dbl_ovrd=off  optimize=Area  use_rpm=off  block_type=sprom
      settings: xl_area=[1 1 0 1 0 0 0]  block_version=VER_STRING_GOES_HERE
  x_dly : Outport
  x_dly_vout : Outport  Port=2
  y : Outport  Port=3
  y_vout : Outport  Port=4
  wires:
  [ltsCorr_ld] -> MAC/3(ld), Delay/1, Gateway Out3/1
  ROM/1 -> MAC/2(coeff), Gateway Out2/1
  MAC/1(yn) -> y/1, Gateway Out4/1
  ASR/1 -> MAC/1(a), x_dly/1, Gateway Out1/1
  [ltsCorr_cAddr] -> ROM/1, Gateway Out5/1
  [ltsCorr_dAddr] -> ASR/2, Gateway Out7/1
  x/1 -> ASR/1, Gateway Out6/1
  vin/1 -> ASR/3, Gateway Out8/1, MAC/4(rst), x_dly_vout/1
  Delay/1 -> y_vout/1
  Gateway Out8/1 -> ?  "X Vin"
  Gateway Out7/1 -> ?  "ASR Addr"
  Gateway Out6/1 -> ?  "X In"
  Gateway Out1/1 -> ?  "ASR Out"
  Gateway Out2/1 -> ?  "ROM Out"
  Gateway Out3/1 -> ?  "Corr Ld"
  Gateway Out4/1 -> ?  "Corr Out"
  Gateway Out5/1 -> ?  "ROM Addr"

== system wlan_phy_rx_pmd/Sync & Antenna Sel/LTS Correlation/Correlator/C5/C7 ==
  x : Inport
  vin : Inport  Port=2
  "  " : Inport  Port=3
  "     " : Inport  Port=4
  ASR : xbsIndex_r4/Addressable Shift Register  infer_latency=off  depth=length(coef2)  initVector=[0]  en=on  dbl_ovrd=off  use_behavioral_HDL=off  Optimization=Resource  use_rpm=off  block_type=addrsr
      settings: infoedit="Delay of configurable length.  Any element in the delay line can be addressed and driven onto the output port.<br><br>Hardware notes: This block is implemented using SRL16s. If Virtex-4 or Spartan-3 device is used, multiple SRLC16s are cascaded together."  xl_area=[1 0 0 2 0 0 0]  block_version=VER_STRING_GOES_HERE
  Delay : xbsIndex_r4/Delay  rst=off  infoeditControl=Selection of Reset will increase slice count due to use of real FFs and instead of SRLs  en=off  latency=1  dbl_ovrd=off  reg_retiming=off  block_type=delay
  Gateway Out{1..8} (8 alike) : xbsIndex_r4/Gateway Out  inherit_from_input=off  hdl_port=off  timing_constraint=None  locs_specified=off  LOCs={}  UseAsDAC=off  DACChannel='1'  block_type=gatewayout
      settings: infoedit="Gateway out block.  Converts Xilinx fixed point inputs into ouputs of type Simulink integer, double, or fixed point.<P><P>Hardware notes:  In hardware these blocks become top level output ports or are discarded, depending on how they are configured."  xl_area=[0 0 0 0 0 0 0]  block_version=VER_STRING_GOES_HERE
  MAC : Subsystem
  ROM : xbsIndex_r4/ROM  depth=length(coef2)  initVector=coef2  distributed_mem=Distributed memory  rst=off  init_reg=0  en=off  latency=1  gui_display_data_type=Fixed-point  arith_type="Signed  (2's comp)"  n_bits=longCorr_coef_nbits  bin_pt=longCorr_coef_bp  preci_type=Single  exp_width=8  frac_width=24  dbl_ovrd=off  optimize=Area  use_rpm=off  block_type=sprom
      settings: xl_area=[1 1 0 1 0 0 0]  block_version=VER_STRING_GOES_HERE
  x_dly : Outport
  x_dly_vout : Outport  Port=2
  y : Outport  Port=3
  y_vout : Outport  Port=4
  wires:
  Gateway Out5/1 -> ?  "ROM Addr"
  Gateway Out4/1 -> ?  "Corr Out"
  Gateway Out3/1 -> ?  "Corr Ld"
  Gateway Out2/1 -> ?  "ROM Out"
  Gateway Out1/1 -> ?  "ASR Out"
  Gateway Out6/1 -> ?  "X In"
  Gateway Out7/1 -> ?  "ASR Addr"
  Gateway Out8/1 -> ?  "X Vin"
  Delay/1 -> y_vout/1
  vin/1 -> x_dly_vout/1, MAC/4(rst), Gateway Out8/1, ASR/3
  x/1 -> Gateway Out6/1, ASR/1
  [ltsCorr_dAddr] -> Gateway Out7/1, ASR/2
  [ltsCorr_cAddr] -> Gateway Out5/1, ROM/1
  ASR/1 -> Gateway Out1/1, x_dly/1, MAC/1(a)
  MAC/1(yn) -> Gateway Out4/1, y/1
  ROM/1 -> Gateway Out2/1, MAC/2(coeff)
  [ltsCorr_ld] -> Gateway Out3/1, Delay/1, MAC/3(ld)

== system wlan_phy_rx_pmd/Sync & Antenna Sel/LTS Correlation/Correlator/C5/C8 ==
  x : Inport
  vin : Inport  Port=2
  "  " : Inport  Port=3
  "     " : Inport  Port=4
  ASR : xbsIndex_r4/Addressable Shift Register  infer_latency=off  depth=length(coef2)  initVector=[0]  en=on  dbl_ovrd=off  use_behavioral_HDL=off  Optimization=Resource  use_rpm=off  block_type=addrsr
      settings: infoedit="Delay of configurable length.  Any element in the delay line can be addressed and driven onto the output port.<br><br>Hardware notes: This block is implemented using SRL16s. If Virtex-4 or Spartan-3 device is used, multiple SRLC16s are cascaded together."  xl_area=[1 0 0 2 0 0 0]  block_version=VER_STRING_GOES_HERE
  Delay : xbsIndex_r4/Delay  rst=off  infoeditControl=Selection of Reset will increase slice count due to use of real FFs and instead of SRLs  en=off  latency=1  dbl_ovrd=off  reg_retiming=off  block_type=delay
  Gateway Out{1..8} (8 alike) : xbsIndex_r4/Gateway Out  inherit_from_input=off  hdl_port=off  timing_constraint=None  locs_specified=off  LOCs={}  UseAsDAC=off  DACChannel='1'  block_type=gatewayout
      settings: infoedit="Gateway out block.  Converts Xilinx fixed point inputs into ouputs of type Simulink integer, double, or fixed point.<P><P>Hardware notes:  In hardware these blocks become top level output ports or are discarded, depending on how they are configured."  xl_area=[0 0 0 0 0 0 0]  block_version=VER_STRING_GOES_HERE
  MAC : Subsystem
  ROM : xbsIndex_r4/ROM  depth=length(coef2)  initVector=coef2  distributed_mem=Distributed memory  rst=off  init_reg=0  en=off  latency=1  gui_display_data_type=Fixed-point  arith_type="Signed  (2's comp)"  n_bits=longCorr_coef_nbits  bin_pt=longCorr_coef_bp  preci_type=Single  exp_width=8  frac_width=24  dbl_ovrd=off  optimize=Area  use_rpm=off  block_type=sprom
      settings: xl_area=[1 1 0 1 0 0 0]  block_version=VER_STRING_GOES_HERE
  x_dly : Outport
  x_dly_vout : Outport  Port=2
  y : Outport  Port=3
  y_vout : Outport  Port=4
  wires:
  [ltsCorr_ld] -> MAC/3(ld), Delay/1, Gateway Out3/1
  ROM/1 -> MAC/2(coeff), Gateway Out2/1
  MAC/1(yn) -> y/1, Gateway Out4/1
  ASR/1 -> MAC/1(a), x_dly/1, Gateway Out1/1
  [ltsCorr_cAddr] -> ROM/1, Gateway Out5/1
  [ltsCorr_dAddr] -> ASR/2, Gateway Out7/1
  x/1 -> ASR/1, Gateway Out6/1
  vin/1 -> ASR/3, Gateway Out8/1, MAC/4(rst), x_dly_vout/1
  Delay/1 -> y_vout/1
  Gateway Out8/1 -> ?  "X Vin"
  Gateway Out7/1 -> ?  "ASR Addr"
  Gateway Out6/1 -> ?  "X In"
  Gateway Out1/1 -> ?  "ASR Out"
  Gateway Out2/1 -> ?  "ROM Out"
  Gateway Out3/1 -> ?  "Corr Ld"
  Gateway Out4/1 -> ?  "Corr Out"
  Gateway Out5/1 -> ?  "ROM Addr"

== system wlan_phy_rx_pmd/Sync & Antenna Sel/LTS Correlation/Correlator/C6/C1 ==
  x : Inport
  vin : Inport  Port=2
  "  " : Inport  Port=3
  "     " : Inport  Port=4
  ASR : xbsIndex_r4/Addressable Shift Register  infer_latency=off  depth=length(coef2)  initVector=[0]  en=on  dbl_ovrd=off  use_behavioral_HDL=off  Optimization=Resource  use_rpm=off  block_type=addrsr
      settings: infoedit="Delay of configurable length.  Any element in the delay line can be addressed and driven onto the output port.<br><br>Hardware notes: This block is implemented using SRL16s. If Virtex-4 or Spartan-3 device is used, multiple SRLC16s are cascaded together."  xl_area=[1 0 0 2 0 0 0]  block_version=VER_STRING_GOES_HERE
  Delay : xbsIndex_r4/Delay  rst=off  infoeditControl=Selection of Reset will increase slice count due to use of real FFs and instead of SRLs  en=off  latency=1  dbl_ovrd=off  reg_retiming=off  block_type=delay
  Gateway Out{1..8} (8 alike) : xbsIndex_r4/Gateway Out  inherit_from_input=off  hdl_port=off  timing_constraint=None  locs_specified=off  LOCs={}  UseAsDAC=off  DACChannel='1'  block_type=gatewayout
      settings: infoedit="Gateway out block.  Converts Xilinx fixed point inputs into ouputs of type Simulink integer, double, or fixed point.<P><P>Hardware notes:  In hardware these blocks become top level output ports or are discarded, depending on how they are configured."  xl_area=[0 0 0 0 0 0 0]  block_version=VER_STRING_GOES_HERE
  MAC : Subsystem
  ROM : xbsIndex_r4/ROM  depth=length(coef2)  initVector=coef2  distributed_mem=Distributed memory  rst=off  init_reg=0  en=off  latency=1  gui_display_data_type=Fixed-point  arith_type="Signed  (2's comp)"  n_bits=longCorr_coef_nbits  bin_pt=longCorr_coef_bp  preci_type=Single  exp_width=8  frac_width=24  dbl_ovrd=off  optimize=Area  use_rpm=off  block_type=sprom
      settings: xl_area=[1 1 0 1 0 0 0]  block_version=VER_STRING_GOES_HERE
  x_dly : Outport
  x_dly_vout : Outport  Port=2
  y : Outport  Port=3
  y_vout : Outport  Port=4
  wires:
  [ltsCorr_ld] -> MAC/3(ld), Delay/1, Gateway Out3/1
  ROM/1 -> MAC/2(coeff), Gateway Out2/1
  MAC/1(yn) -> y/1, Gateway Out4/1
  ASR/1 -> MAC/1(a), x_dly/1, Gateway Out1/1
  [ltsCorr_cAddr] -> ROM/1, Gateway Out5/1
  [ltsCorr_dAddr] -> ASR/2, Gateway Out7/1
  x/1 -> ASR/1, Gateway Out6/1
  vin/1 -> ASR/3, Gateway Out8/1, MAC/4(rst), x_dly_vout/1
  Delay/1 -> y_vout/1
  Gateway Out8/1 -> ?  "X Vin"
  Gateway Out7/1 -> ?  "ASR Addr"
  Gateway Out6/1 -> ?  "X In"
  Gateway Out1/1 -> ?  "ASR Out"
  Gateway Out2/1 -> ?  "ROM Out"
  Gateway Out3/1 -> ?  "Corr Ld"
  Gateway Out4/1 -> ?  "Corr Out"
  Gateway Out5/1 -> ?  "ROM Addr"

== system wlan_phy_rx_pmd/Sync & Antenna Sel/LTS Correlation/Correlator/C6/C2 ==
  x : Inport
  vin : Inport  Port=2
  "  " : Inport  Port=3
  "     " : Inport  Port=4
  ASR : xbsIndex_r4/Addressable Shift Register  infer_latency=off  depth=length(coef2)  initVector=[0]  en=on  dbl_ovrd=off  use_behavioral_HDL=off  Optimization=Resource  use_rpm=off  block_type=addrsr
      settings: infoedit="Delay of configurable length.  Any element in the delay line can be addressed and driven onto the output port.<br><br>Hardware notes: This block is implemented using SRL16s. If Virtex-4 or Spartan-3 device is used, multiple SRLC16s are cascaded together."  xl_area=[1 0 0 2 0 0 0]  block_version=VER_STRING_GOES_HERE
  Delay : xbsIndex_r4/Delay  rst=off  infoeditControl=Selection of Reset will increase slice count due to use of real FFs and instead of SRLs  en=off  latency=1  dbl_ovrd=off  reg_retiming=off  block_type=delay
  Gateway Out{1..8} (8 alike) : xbsIndex_r4/Gateway Out  inherit_from_input=off  hdl_port=off  timing_constraint=None  locs_specified=off  LOCs={}  UseAsDAC=off  DACChannel='1'  block_type=gatewayout
      settings: infoedit="Gateway out block.  Converts Xilinx fixed point inputs into ouputs of type Simulink integer, double, or fixed point.<P><P>Hardware notes:  In hardware these blocks become top level output ports or are discarded, depending on how they are configured."  xl_area=[0 0 0 0 0 0 0]  block_version=VER_STRING_GOES_HERE
  MAC : Subsystem
  ROM : xbsIndex_r4/ROM  depth=length(coef2)  initVector=coef2  distributed_mem=Distributed memory  rst=off  init_reg=0  en=off  latency=1  gui_display_data_type=Fixed-point  arith_type="Signed  (2's comp)"  n_bits=longCorr_coef_nbits  bin_pt=longCorr_coef_bp  preci_type=Single  exp_width=8  frac_width=24  dbl_ovrd=off  optimize=Area  use_rpm=off  block_type=sprom
      settings: xl_area=[1 1 0 1 0 0 0]  block_version=VER_STRING_GOES_HERE
  x_dly : Outport
  x_dly_vout : Outport  Port=2
  y : Outport  Port=3
  y_vout : Outport  Port=4
  wires:
  Gateway Out5/1 -> ?  "ROM Addr"
  Gateway Out4/1 -> ?  "Corr Out"
  Gateway Out3/1 -> ?  "Corr Ld"
  Gateway Out2/1 -> ?  "ROM Out"
  Gateway Out1/1 -> ?  "ASR Out"
  Gateway Out6/1 -> ?  "X In"
  Gateway Out7/1 -> ?  "ASR Addr"
  Gateway Out8/1 -> ?  "X Vin"
  Delay/1 -> y_vout/1
  vin/1 -> x_dly_vout/1, MAC/4(rst), Gateway Out8/1, ASR/3
  x/1 -> Gateway Out6/1, ASR/1
  [ltsCorr_dAddr] -> Gateway Out7/1, ASR/2
  [ltsCorr_cAddr] -> Gateway Out5/1, ROM/1
  ASR/1 -> Gateway Out1/1, x_dly/1, MAC/1(a)
  MAC/1(yn) -> Gateway Out4/1, y/1
  ROM/1 -> Gateway Out2/1, MAC/2(coeff)
  [ltsCorr_ld] -> Gateway Out3/1, Delay/1, MAC/3(ld)

== system wlan_phy_rx_pmd/Sync & Antenna Sel/LTS Correlation/Correlator/C6/C3 ==
  x : Inport
  vin : Inport  Port=2
  "  " : Inport  Port=3
  "     " : Inport  Port=4
  ASR : xbsIndex_r4/Addressable Shift Register  infer_latency=off  depth=length(coef2)  initVector=[0]  en=on  dbl_ovrd=off  use_behavioral_HDL=off  Optimization=Resource  use_rpm=off  block_type=addrsr
      settings: infoedit="Delay of configurable length.  Any element in the delay line can be addressed and driven onto the output port.<br><br>Hardware notes: This block is implemented using SRL16s. If Virtex-4 or Spartan-3 device is used, multiple SRLC16s are cascaded together."  xl_area=[1 0 0 2 0 0 0]  block_version=VER_STRING_GOES_HERE
  Delay : xbsIndex_r4/Delay  rst=off  infoeditControl=Selection of Reset will increase slice count due to use of real FFs and instead of SRLs  en=off  latency=1  dbl_ovrd=off  reg_retiming=off  block_type=delay
  Gateway Out{1..8} (8 alike) : xbsIndex_r4/Gateway Out  inherit_from_input=off  hdl_port=off  timing_constraint=None  locs_specified=off  LOCs={}  UseAsDAC=off  DACChannel='1'  block_type=gatewayout
      settings: infoedit="Gateway out block.  Converts Xilinx fixed point inputs into ouputs of type Simulink integer, double, or fixed point.<P><P>Hardware notes:  In hardware these blocks become top level output ports or are discarded, depending on how they are configured."  xl_area=[0 0 0 0 0 0 0]  block_version=VER_STRING_GOES_HERE
  MAC : Subsystem
  ROM : xbsIndex_r4/ROM  depth=length(coef2)  initVector=coef2  distributed_mem=Distributed memory  rst=off  init_reg=0  en=off  latency=1  gui_display_data_type=Fixed-point  arith_type="Signed  (2's comp)"  n_bits=longCorr_coef_nbits  bin_pt=longCorr_coef_bp  preci_type=Single  exp_width=8  frac_width=24  dbl_ovrd=off  optimize=Area  use_rpm=off  block_type=sprom
      settings: xl_area=[1 1 0 1 0 0 0]  block_version=VER_STRING_GOES_HERE
  x_dly : Outport
  x_dly_vout : Outport  Port=2
  y : Outport  Port=3
  y_vout : Outport  Port=4
  wires:
  [ltsCorr_ld] -> MAC/3(ld), Delay/1, Gateway Out3/1
  ROM/1 -> MAC/2(coeff), Gateway Out2/1
  MAC/1(yn) -> y/1, Gateway Out4/1
  ASR/1 -> MAC/1(a), x_dly/1, Gateway Out1/1
  [ltsCorr_cAddr] -> ROM/1, Gateway Out5/1
  [ltsCorr_dAddr] -> ASR/2, Gateway Out7/1
  x/1 -> ASR/1, Gateway Out6/1
  vin/1 -> ASR/3, Gateway Out8/1, MAC/4(rst), x_dly_vout/1
  Delay/1 -> y_vout/1
  Gateway Out8/1 -> ?  "X Vin"
  Gateway Out7/1 -> ?  "ASR Addr"
  Gateway Out6/1 -> ?  "X In"
  Gateway Out1/1 -> ?  "ASR Out"
  Gateway Out2/1 -> ?  "ROM Out"
  Gateway Out3/1 -> ?  "Corr Ld"
  Gateway Out4/1 -> ?  "Corr Out"
  Gateway Out5/1 -> ?  "ROM Addr"

== system wlan_phy_rx_pmd/Sync & Antenna Sel/LTS Correlation/Correlator/C6/C4 ==
  x : Inport
  vin : Inport  Port=2
  "  " : Inport  Port=3
  "     " : Inport  Port=4
  ASR : xbsIndex_r4/Addressable Shift Register  infer_latency=off  depth=length(coef2)  initVector=[0]  en=on  dbl_ovrd=off  use_behavioral_HDL=off  Optimization=Resource  use_rpm=off  block_type=addrsr
      settings: infoedit="Delay of configurable length.  Any element in the delay line can be addressed and driven onto the output port.<br><br>Hardware notes: This block is implemented using SRL16s. If Virtex-4 or Spartan-3 device is used, multiple SRLC16s are cascaded together."  xl_area=[1 0 0 2 0 0 0]  block_version=VER_STRING_GOES_HERE
  Delay : xbsIndex_r4/Delay  rst=off  infoeditControl=Selection of Reset will increase slice count due to use of real FFs and instead of SRLs  en=off  latency=1  dbl_ovrd=off  reg_retiming=off  block_type=delay
  Gateway Out{1..8} (8 alike) : xbsIndex_r4/Gateway Out  inherit_from_input=off  hdl_port=off  timing_constraint=None  locs_specified=off  LOCs={}  UseAsDAC=off  DACChannel='1'  block_type=gatewayout
      settings: infoedit="Gateway out block.  Converts Xilinx fixed point inputs into ouputs of type Simulink integer, double, or fixed point.<P><P>Hardware notes:  In hardware these blocks become top level output ports or are discarded, depending on how they are configured."  xl_area=[0 0 0 0 0 0 0]  block_version=VER_STRING_GOES_HERE
  MAC : Subsystem
  ROM : xbsIndex_r4/ROM  depth=length(coef2)  initVector=coef2  distributed_mem=Distributed memory  rst=off  init_reg=0  en=off  latency=1  gui_display_data_type=Fixed-point  arith_type="Signed  (2's comp)"  n_bits=longCorr_coef_nbits  bin_pt=longCorr_coef_bp  preci_type=Single  exp_width=8  frac_width=24  dbl_ovrd=off  optimize=Area  use_rpm=off  block_type=sprom
      settings: xl_area=[1 1 0 1 0 0 0]  block_version=VER_STRING_GOES_HERE
  x_dly : Outport
  x_dly_vout : Outport  Port=2
  y : Outport  Port=3
  y_vout : Outport  Port=4
  wires:
  Gateway Out5/1 -> ?  "ROM Addr"
  Gateway Out4/1 -> ?  "Corr Out"
  Gateway Out3/1 -> ?  "Corr Ld"
  Gateway Out2/1 -> ?  "ROM Out"
  Gateway Out1/1 -> ?  "ASR Out"
  Gateway Out6/1 -> ?  "X In"
  Gateway Out7/1 -> ?  "ASR Addr"
  Gateway Out8/1 -> ?  "X Vin"
  Delay/1 -> y_vout/1
  vin/1 -> x_dly_vout/1, MAC/4(rst), Gateway Out8/1, ASR/3
  x/1 -> Gateway Out6/1, ASR/1
  [ltsCorr_dAddr] -> Gateway Out7/1, ASR/2
  [ltsCorr_cAddr] -> Gateway Out5/1, ROM/1
  ASR/1 -> Gateway Out1/1, x_dly/1, MAC/1(a)
  MAC/1(yn) -> Gateway Out4/1, y/1
  ROM/1 -> Gateway Out2/1, MAC/2(coeff)
  [ltsCorr_ld] -> Gateway Out3/1, Delay/1, MAC/3(ld)

== system wlan_phy_rx_pmd/Sync & Antenna Sel/LTS Correlation/Correlator/C6/C5 ==
  x : Inport
  vin : Inport  Port=2
  "  " : Inport  Port=3
  "     " : Inport  Port=4
  ASR : xbsIndex_r4/Addressable Shift Register  infer_latency=off  depth=length(coef2)  initVector=[0]  en=on  dbl_ovrd=off  use_behavioral_HDL=off  Optimization=Resource  use_rpm=off  block_type=addrsr
      settings: infoedit="Delay of configurable length.  Any element in the delay line can be addressed and driven onto the output port.<br><br>Hardware notes: This block is implemented using SRL16s. If Virtex-4 or Spartan-3 device is used, multiple SRLC16s are cascaded together."  xl_area=[1 0 0 2 0 0 0]  block_version=VER_STRING_GOES_HERE
  Delay : xbsIndex_r4/Delay  rst=off  infoeditControl=Selection of Reset will increase slice count due to use of real FFs and instead of SRLs  en=off  latency=1  dbl_ovrd=off  reg_retiming=off  block_type=delay
  Gateway Out{1..8} (8 alike) : xbsIndex_r4/Gateway Out  inherit_from_input=off  hdl_port=off  timing_constraint=None  locs_specified=off  LOCs={}  UseAsDAC=off  DACChannel='1'  block_type=gatewayout
      settings: infoedit="Gateway out block.  Converts Xilinx fixed point inputs into ouputs of type Simulink integer, double, or fixed point.<P><P>Hardware notes:  In hardware these blocks become top level output ports or are discarded, depending on how they are configured."  xl_area=[0 0 0 0 0 0 0]  block_version=VER_STRING_GOES_HERE
  MAC : Subsystem
  ROM : xbsIndex_r4/ROM  depth=length(coef2)  initVector=coef2  distributed_mem=Distributed memory  rst=off  init_reg=0  en=off  latency=1  gui_display_data_type=Fixed-point  arith_type="Signed  (2's comp)"  n_bits=longCorr_coef_nbits  bin_pt=longCorr_coef_bp  preci_type=Single  exp_width=8  frac_width=24  dbl_ovrd=off  optimize=Area  use_rpm=off  block_type=sprom
      settings: xl_area=[1 1 0 1 0 0 0]  block_version=VER_STRING_GOES_HERE
  x_dly : Outport
  x_dly_vout : Outport  Port=2
  y : Outport  Port=3
  y_vout : Outport  Port=4
  wires:
  [ltsCorr_ld] -> MAC/3(ld), Delay/1, Gateway Out3/1
  ROM/1 -> MAC/2(coeff), Gateway Out2/1
  MAC/1(yn) -> y/1, Gateway Out4/1
  ASR/1 -> MAC/1(a), x_dly/1, Gateway Out1/1
  [ltsCorr_cAddr] -> ROM/1, Gateway Out5/1
  [ltsCorr_dAddr] -> ASR/2, Gateway Out7/1
  x/1 -> ASR/1, Gateway Out6/1
  vin/1 -> ASR/3, Gateway Out8/1, MAC/4(rst), x_dly_vout/1
  Delay/1 -> y_vout/1
  Gateway Out8/1 -> ?  "X Vin"
  Gateway Out7/1 -> ?  "ASR Addr"
  Gateway Out6/1 -> ?  "X In"
  Gateway Out1/1 -> ?  "ASR Out"
  Gateway Out2/1 -> ?  "ROM Out"
  Gateway Out3/1 -> ?  "Corr Ld"
  Gateway Out4/1 -> ?  "Corr Out"
  Gateway Out5/1 -> ?  "ROM Addr"
